simulink model "wlan_phy_rx_pmd"  (Simulink 7.8) (continued)
== system wlan_phy_rx_pmd/MAC Outputs == (continued)
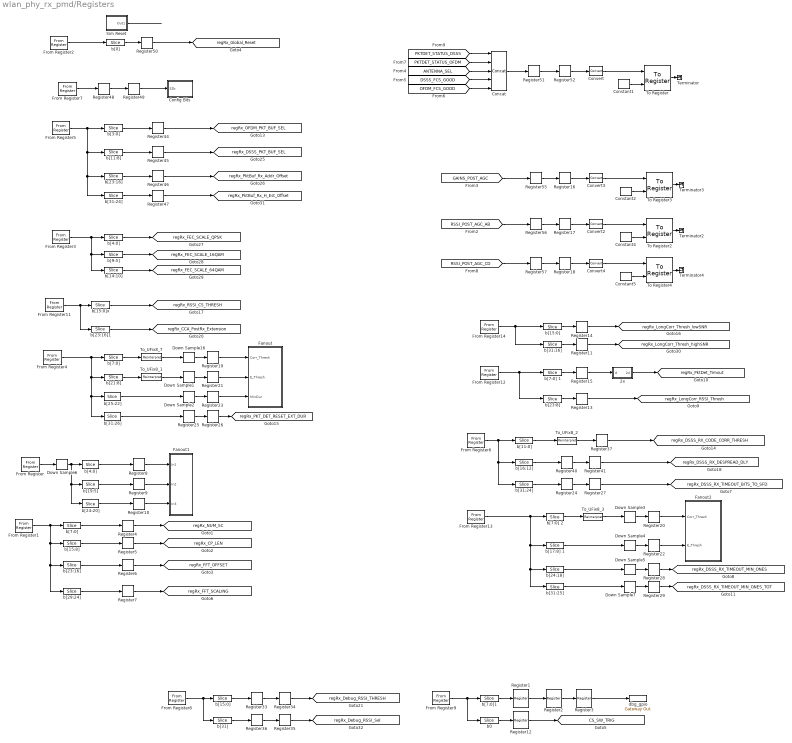
== system wlan_phy_rx_pmd/Registers ==
  " b[23:16] " : xbsIndex_r4/Slice  nbits=8  boolean_output=off  mode=Lower Bit Location + Width  bit1=0  base1=MSB of Input  bit0=16  base0=LSB of Input  dbl_ovrd=off  block_type=slice
      settings: block_version=VER_STRING_GOES_HERE
  " b[31:24]" : xbsIndex_r4/Slice  nbits=8  boolean_output=off  mode=Lower Bit Location + Width  bit1=0  base1=MSB of Input  bit0=24  base0=LSB of Input  dbl_ovrd=off  block_type=slice
      settings: block_version=VER_STRING_GOES_HERE
  "2x " : Subsystem
  Concat : xbsIndex_r4/Concat  num_inputs=5  dbl_ovrd=off  block_type=concat
      settings: block_version=8.2.02
  Config Bits : Subsystem
  Constant{1..2,4..5} (4 alike) : xbsIndex_r4/Constant  const=1  gui_display_data_type=Boolean  arith_type=Boolean  n_bits=30  bin_pt=0  preci_type=Single  exp_width=8  frac_width=24  explicit_period=on  period=1  equ=P=C  opselect=C  inp2=PCIN>>17  opr=+  inp1=P  carry=CIN  dbl_ovrd=off  block_type=constant
  Convert{,2..4} (4 alike) : xbsIndex_r4/Convert  gui_display_data_type=Fixed-point  arith_type=Unsigned  n_bits=32  bin_pt=0  float_type=Single  exp_bits=8  fraction_bits=24  quantization=Truncate  overflow=Wrap  en=off  latency=0  dbl_ovrd=off  pipeline=off  block_type=convert
  Down Sample{1..5,7,16} (7 alike) : xbsIndex_r4/Down Sample  sample_ratio=8  sample_phase="Last Value of Frame  (most efficient)"  en=off  latency=1  dbl_ovrd=off  block_type=dsamp
      settings: block_version=VER_STRING_GOES_HERE
  Down Sample6 : xbsIndex_r4/Down Sample  sample_ratio=16  sample_phase="Last Value of Frame  (most efficient)"  en=off  latency=1  dbl_ovrd=off  block_type=dsamp
      settings: block_version=VER_STRING_GOES_HERE
  Fanout : Subsystem
  Fanout1 : Subsystem
  Fanout2 : Subsystem
  From Register : xbsIndex_r4/From Register  shared_memory_name='PKTDET_RSSI_CONFIG'  init=REG_RX_PKTDET_RSSI_CONFIG  period=1  ownership=Locally owned and initialized  gui_display_data_type=Fixed-point  arith_type=Unsigned  n_bits=32  bin_pt=0  preci_type=Single  dbl_ovrd=off  block_type=fromreg
      settings: AttributesFormatString=<< %<shared_memory_name> >>  infoedit="Register block that reads data to a shared memory register.  Delay of one sample period."
  From Register1 : xbsIndex_r4/From Register  shared_memory_name='FFT_Config'  init=REG_RX_FFT_Config  period=1  ownership=Locally owned and initialized  gui_display_data_type=Fixed-point  arith_type=Unsigned  n_bits=32  bin_pt=0  preci_type=Single  dbl_ovrd=off  block_type=fromreg
      settings: AttributesFormatString=<< %<shared_memory_name> >>  infoedit="Register block that reads data to a shared memory register.  Delay of one sample period."
  From Register11 : xbsIndex_r4/From Register  shared_memory_name='PHY_CCA_CONFIG'  init=REG_RX_CCA_CONFIG  period=1  ownership=Locally owned and initialized  gui_display_data_type=Fixed-point  arith_type=Unsigned  n_bits=32  bin_pt=0  preci_type=Single  dbl_ovrd=off  block_type=fromreg
      settings: AttributesFormatString=<< %<shared_memory_name> >>  infoedit="Register block that reads data to a shared memory register.  Delay of one sample period."
  From Register12 : xbsIndex_r4/From Register  shared_memory_name='LTS_Corr_Config'  init=REG_RX_LTS_Corr_Confg  period=1  ownership=Locally owned and initialized  gui_display_data_type=Fixed-point  arith_type=Unsigned  n_bits=32  bin_pt=0  preci_type=Single  dbl_ovrd=off  block_type=fromreg
      settings: AttributesFormatString=<< %<shared_memory_name> >>  infoedit="Register block that reads data to a shared memory register.  Delay of one sample period."
  From Register13 : xbsIndex_r4/From Register  shared_memory_name='PKTDET_DSSS_CONFIG'  init=REG_RX_PktDet_DSSS_Config  period=1  ownership=Locally owned and initialized  gui_display_data_type=Fixed-point  arith_type=Unsigned  n_bits=32  bin_pt=0  preci_type=Single  dbl_ovrd=off  block_type=fromreg
      settings: AttributesFormatString=<< %<shared_memory_name> >>  infoedit="Register block that reads data to a shared memory register.  Delay of one sample period."
  From Register14 : xbsIndex_r4/From Register  shared_memory_name='LTS_Corr_Thresh'  init=REG_RX_LTS_Corr_Thresh  period=1  ownership=Locally owned and initialized  gui_display_data_type=Fixed-point  arith_type=Unsigned  n_bits=32  bin_pt=0  preci_type=Single  dbl_ovrd=off  block_type=fromreg
      settings: AttributesFormatString=<< %<shared_memory_name> >>  infoedit="Register block that reads data to a shared memory register.  Delay of one sample period."
  From Register2 : xbsIndex_r4/From Register  shared_memory_name='Control'  init=REG_RX_Control  period=1  ownership=Locally owned and initialized  gui_display_data_type=Fixed-point  arith_type=Unsigned  n_bits=32  bin_pt=0  preci_type=Single  dbl_ovrd=off  block_type=fromreg
      settings: AttributesFormatString=<< %<shared_memory_name> >>  infoedit="Register block that reads data to a shared memory register.  Delay of one sample period."
  From Register3 : xbsIndex_r4/From Register  shared_memory_name='FEC_CONFIG'  init=REG_RX_FEC_Config  period=1  ownership=Locally owned and initialized  gui_display_data_type=Fixed-point  arith_type=Unsigned  n_bits=32  bin_pt=0  preci_type=Single  dbl_ovrd=off  block_type=fromreg
      settings: AttributesFormatString=<< %<shared_memory_name> >>  infoedit="Register block that reads data to a shared memory register.  Delay of one sample period."
  From Register4 : xbsIndex_r4/From Register  shared_memory_name='PKTDET_AUTOCORR_CONFIG'  init=REG_RX_PktDet_AutoCorr_Config  period=1  ownership=Locally owned and initialized  gui_display_data_type=Fixed-point  arith_type=Unsigned  n_bits=32  bin_pt=0  preci_type=Single  dbl_ovrd=off  block_type=fromreg
      settings: AttributesFormatString=<< %<shared_memory_name> >>  infoedit="Register block that reads data to a shared memory register.  Delay of one sample period."
  From Register5 : xbsIndex_r4/From Register  shared_memory_name='PKT_BUF_SEL'  init=REG_RX_PktBuf_Sel  period=1  ownership=Locally owned and initialized  gui_display_data_type=Fixed-point  arith_type=Unsigned  n_bits=32  bin_pt=0  preci_type=Single  dbl_ovrd=off  block_type=fromreg
      settings: AttributesFormatString=<< %<shared_memory_name> >>  infoedit="Register block that reads data to a shared memory register.  Delay of one sample period."
  From Register6 : xbsIndex_r4/From Register  shared_memory_name='DSSS_RX_CONFIG'  init=REG_RX_DSSS_RX_CONFIG  period=1  ownership=Locally owned and initialized  gui_display_data_type=Fixed-point  arith_type=Unsigned  n_bits=32  bin_pt=0  preci_type=Single  dbl_ovrd=off  block_type=fromreg
      settings: AttributesFormatString=<< %<shared_memory_name> >>  infoedit="Register block that reads data to a shared memory register.  Delay of one sample period."
  From Register7 : xbsIndex_r4/From Register  shared_memory_name='CONFIG'  init=REG_RX_Config  period=1  ownership=Locally owned and initialized  gui_display_data_type=Fixed-point  arith_type=Unsigned  n_bits=32  bin_pt=0  preci_type=Single  dbl_ovrd=off  block_type=fromreg
      settings: AttributesFormatString=<< %<shared_memory_name> >>  infoedit="Register block that reads data to a shared memory register.  Delay of one sample period."
  From Register8 : xbsIndex_r4/From Register  shared_memory_name='RSSI_THRESH'  init=600  period=1  ownership=Locally owned and initialized  gui_display_data_type=Fixed-point  arith_type=Unsigned  n_bits=32  bin_pt=0  preci_type=Single  dbl_ovrd=off  block_type=fromreg
      settings: AttributesFormatString=<< %<shared_memory_name> >>  infoedit="Register block that reads data to a shared memory register.  Delay of one sample period."
  From Register9 : xbsIndex_r4/From Register  shared_memory_name='DEBUG_GPIO'  init=0  period=1  ownership=Locally owned and initialized  gui_display_data_type=Fixed-point  arith_type=Unsigned  n_bits=32  bin_pt=0  preci_type=Single  dbl_ovrd=off  block_type=fromreg
      settings: AttributesFormatString=<< %<shared_memory_name> >>  infoedit="Register block that reads data to a shared memory register.  Delay of one sample period."
  Register{1..29,33..37,40..41,44..52,55..57} (48 alike) : xbsIndex_r4/Register  init=0  rst=off  en=off  dbl_ovrd=off  block_type=register
  Sim Reset : Subsystem
  Terminator{,2..4} (4 alike) : Terminator
  To Register : xbsIndex_r4/To Register  shared_memory_name='Status'  init=0  ownership=Locally owned and initialized  explicit_data_type=on  gui_display_data_type=Fixed-point  arith_type=Unsigned  n_bits=32  bin_pt=0  preci_type=Single  dbl_ovrd=off  block_type=toreg
      settings: AttributesFormatString=<< %<shared_memory_name> >>  infoedit="Register block that writes data to a shared memory register.  Delay of one sample period."
  To Register2 : xbsIndex_r4/To Register  shared_memory_name='RX_PKT_RSSI_AB'  init=0  ownership=Locally owned and initialized  explicit_data_type=on  gui_display_data_type=Fixed-point  arith_type=Unsigned  n_bits=32  bin_pt=0  preci_type=Single  dbl_ovrd=off  block_type=toreg
      settings: AttributesFormatString=<< %<shared_memory_name> >>  infoedit="Register block that writes data to a shared memory register.  Delay of one sample period."
  To Register3 : xbsIndex_r4/To Register  shared_memory_name='RX_PKT_AGC_GAINS'  init=0  ownership=Locally owned and initialized  explicit_data_type=on  gui_display_data_type=Fixed-point  arith_type=Unsigned  n_bits=32  bin_pt=0  preci_type=Single  dbl_ovrd=off  block_type=toreg
      settings: AttributesFormatString=<< %<shared_memory_name> >>  infoedit="Register block that writes data to a shared memory register.  Delay of one sample period."
  To Register4 : xbsIndex_r4/To Register  shared_memory_name='RX_PKT_RSSI_CD'  init=0  ownership=Locally owned and initialized  explicit_data_type=on  gui_display_data_type=Fixed-point  arith_type=Unsigned  n_bits=32  bin_pt=0  preci_type=Single  dbl_ovrd=off  block_type=toreg
      settings: AttributesFormatString=<< %<shared_memory_name> >>  infoedit="Register block that writes data to a shared memory register.  Delay of one sample period."
  To_UFix8_1 : xbsIndex_r4/Reinterpret  force_arith_type=on  arith_type=Unsigned  force_bin_pt=on  bin_pt=8  block_type=cast
      settings: block_version=VER_STRING_GOES_HERE
  To_UFix8_2 : xbsIndex_r4/Reinterpret  force_arith_type=on  arith_type=Unsigned  force_bin_pt=on  bin_pt=4  block_type=cast
      settings: block_version=VER_STRING_GOES_HERE
  To_UFix8_3 : xbsIndex_r4/Reinterpret  force_arith_type=on  arith_type=Unsigned  force_bin_pt=on  bin_pt=6  block_type=cast
      settings: block_version=VER_STRING_GOES_HERE
  To_UFix8_7 : xbsIndex_r4/Reinterpret  force_arith_type=on  arith_type=Unsigned  force_bin_pt=on  bin_pt=8  block_type=cast
      settings: block_version=VER_STRING_GOES_HERE
  b0 : xbsIndex_r4/Slice  nbits=1  boolean_output=off  mode=Lower Bit Location + Width  bit1=0  base1=MSB of Input  bit0=0  base0=LSB of Input  dbl_ovrd=off  block_type=slice
      settings: block_version=VER_STRING_GOES_HERE
  b[0] : xbsIndex_r4/Slice  nbits=1  boolean_output=off  mode=Lower Bit Location + Width  bit1=0  base1=MSB of Input  bit0=0  base0=LSB of Input  dbl_ovrd=off  block_type=slice
      settings: block_version=VER_STRING_GOES_HERE
  "b[11:0]   " : xbsIndex_r4/Slice  nbits=12  boolean_output=off  mode=Lower Bit Location + Width  bit1=0  base1=MSB of Input  bit0=0  base0=LSB of Input  dbl_ovrd=off  block_type=slice
      settings: block_version=VER_STRING_GOES_HERE
  b[11:8] : xbsIndex_r4/Slice  nbits=4  boolean_output=off  mode=Lower Bit Location + Width  bit1=0  base1=MSB of Input  bit0=8  base0=LSB of Input  dbl_ovrd=off  block_type=slice
      settings: block_version=VER_STRING_GOES_HERE
  b[14:10] : xbsIndex_r4/Slice  nbits=5  boolean_output=off  mode=Lower Bit Location + Width  bit1=0  base1=MSB of Input  bit0=10  base0=LSB of Input  dbl_ovrd=off  block_type=slice
      settings: block_version=VER_STRING_GOES_HERE
  b[15:0] : xbsIndex_r4/Slice  nbits=16  boolean_output=off  mode=Lower Bit Location + Width  bit1=0  base1=MSB of Input  bit0=0  base0=LSB of Input  dbl_ovrd=off  block_type=slice
      settings: block_version=VER_STRING_GOES_HERE
  "b[15:0]    " : xbsIndex_r4/Slice  nbits=16  boolean_output=off  mode=Lower Bit Location + Width  bit1=0  base1=MSB of Input  bit0=0  base0=LSB of Input  dbl_ovrd=off  block_type=slice
      settings: block_version=VER_STRING_GOES_HERE
  b[15:0]x : xbsIndex_r4/Slice  nbits=16  boolean_output=off  mode=Lower Bit Location + Width  bit1=0  base1=MSB of Input  bit0=0  base0=LSB of Input  dbl_ovrd=off  block_type=slice
      settings: block_version=VER_STRING_GOES_HERE
  b[15:8] : xbsIndex_r4/Slice  nbits=8  boolean_output=off  mode=Lower Bit Location + Width  bit1=0  base1=MSB of Input  bit0=8  base0=LSB of Input  dbl_ovrd=off  block_type=slice
      settings: block_version=VER_STRING_GOES_HERE
  "b[16:12]   " : xbsIndex_r4/Slice  nbits=5  boolean_output=off  mode=Lower Bit Location + Width  bit1=0  base1=MSB of Input  bit0=12  base0=LSB of Input  dbl_ovrd=off  block_type=slice
      settings: block_version=VER_STRING_GOES_HERE
  b[17:8] 1 : xbsIndex_r4/Slice  nbits=10  boolean_output=off  mode=Lower Bit Location + Width  bit1=0  base1=MSB of Input  bit0=8  base0=LSB of Input  dbl_ovrd=off  block_type=slice
      settings: block_version=VER_STRING_GOES_HERE
  b[19:5] : xbsIndex_r4/Slice  nbits=15  boolean_output=off  mode=Lower Bit Location + Width  bit1=0  base1=MSB of Input  bit0=5  base0=LSB of Input  dbl_ovrd=off  block_type=slice
      settings: block_version=VER_STRING_GOES_HERE
  "b[21:8] " : xbsIndex_r4/Slice  nbits=14  boolean_output=off  mode=Lower Bit Location + Width  bit1=0  base1=MSB of Input  bit0=8  base0=LSB of Input  dbl_ovrd=off  block_type=slice
      settings: block_version=VER_STRING_GOES_HERE
  b[23:16] : xbsIndex_r4/Slice  nbits=8  boolean_output=off  mode=Lower Bit Location + Width  bit1=0  base1=MSB of Input  bit0=16  base0=LSB of Input  dbl_ovrd=off  block_type=slice
      settings: block_version=VER_STRING_GOES_HERE
  b[23:16]1 : xbsIndex_r4/Slice  nbits=8  boolean_output=off  mode=Lower Bit Location + Width  bit1=0  base1=MSB of Input  bit0=16  base0=LSB of Input  dbl_ovrd=off  block_type=slice
      settings: block_version=VER_STRING_GOES_HERE
  b[23:8] : xbsIndex_r4/Slice  nbits=16  boolean_output=off  mode=Lower Bit Location + Width  bit1=0  base1=MSB of Input  bit0=8  base0=LSB of Input  dbl_ovrd=off  block_type=slice
      settings: block_version=VER_STRING_GOES_HERE
  b[24:18] : xbsIndex_r4/Slice  nbits=7  boolean_output=off  mode=Lower Bit Location + Width  bit1=0  base1=MSB of Input  bit0=18  base0=LSB of Input  dbl_ovrd=off  block_type=slice
      settings: block_version=VER_STRING_GOES_HERE
  b[24:20] : xbsIndex_r4/Slice  nbits=5  boolean_output=off  mode=Lower Bit Location + Width  bit1=0  base1=MSB of Input  bit0=20  base0=LSB of Input  dbl_ovrd=off  block_type=slice
      settings: block_version=VER_STRING_GOES_HERE
  "b[25:22] " : xbsIndex_r4/Slice  nbits=4  boolean_output=off  mode=Lower Bit Location + Width  bit1=0  base1=MSB of Input  bit0=22  base0=LSB of Input  dbl_ovrd=off  block_type=slice
      settings: block_version=VER_STRING_GOES_HERE
  b[29:24] : xbsIndex_r4/Slice  nbits=6  boolean_output=off  mode=Lower Bit Location + Width  bit1=0  base1=MSB of Input  bit0=24  base0=LSB of Input  dbl_ovrd=off  block_type=slice
      settings: block_version=VER_STRING_GOES_HERE
  b[31:16] : xbsIndex_r4/Slice  nbits=16  boolean_output=off  mode=Lower Bit Location + Width  bit1=0  base1=MSB of Input  bit0=16  base0=LSB of Input  dbl_ovrd=off  block_type=slice
      settings: block_version=VER_STRING_GOES_HERE
  b[31:24] : xbsIndex_r4/Slice  nbits=8  boolean_output=off  mode=Lower Bit Location + Width  bit1=0  base1=MSB of Input  bit0=24  base0=LSB of Input  dbl_ovrd=off  block_type=slice
      settings: block_version=VER_STRING_GOES_HERE
  b[31:25] : xbsIndex_r4/Slice  nbits=7  boolean_output=off  mode=Lower Bit Location + Width  bit1=0  base1=MSB of Input  bit0=25  base0=LSB of Input  dbl_ovrd=off  block_type=slice
      settings: block_version=VER_STRING_GOES_HERE
  b[31:26] : xbsIndex_r4/Slice  nbits=6  boolean_output=off  mode=Lower Bit Location + Width  bit1=0  base1=MSB of Input  bit0=26  base0=LSB of Input  dbl_ovrd=off  block_type=slice
      settings: block_version=VER_STRING_GOES_HERE
  b[31] : xbsIndex_r4/Slice  nbits=1  boolean_output=off  mode=Lower Bit Location + Width  bit1=0  base1=MSB of Input  bit0=31  base0=LSB of Input  dbl_ovrd=off  block_type=slice
      settings: block_version=VER_STRING_GOES_HERE
  b[3:0] : xbsIndex_r4/Slice  nbits=4  boolean_output=off  mode=Lower Bit Location + Width  bit1=0  base1=MSB of Input  bit0=0  base0=LSB of Input  dbl_ovrd=off  block_type=slice
      settings: block_version=VER_STRING_GOES_HERE
  b[4:0] : xbsIndex_r4/Slice  nbits=5  boolean_output=off  mode=Lower Bit Location + Width  bit1=0  base1=MSB of Input  bit0=0  base0=LSB of Input  dbl_ovrd=off  block_type=slice
      settings: block_version=VER_STRING_GOES_HERE
  "b[4:0]  " : xbsIndex_r4/Slice  nbits=5  boolean_output=off  mode=Lower Bit Location + Width  bit1=0  base1=MSB of Input  bit0=0  base0=LSB of Input  dbl_ovrd=off  block_type=slice
      settings: block_version=VER_STRING_GOES_HERE
  b[7:0] : xbsIndex_r4/Slice  nbits=8  boolean_output=off  mode=Lower Bit Location + Width  bit1=0  base1=MSB of Input  bit0=0  base0=LSB of Input  dbl_ovrd=off  block_type=slice
      settings: block_version=VER_STRING_GOES_HERE
  "b[7:0] " : xbsIndex_r4/Slice  nbits=8  boolean_output=off  mode=Lower Bit Location + Width  bit1=0  base1=MSB of Input  bit0=0  base0=LSB of Input  dbl_ovrd=off  block_type=slice
      settings: block_version=VER_STRING_GOES_HERE
  b[7:0] 1 : xbsIndex_r4/Slice  nbits=8  boolean_output=off  mode=Lower Bit Location + Width  bit1=0  base1=MSB of Input  bit0=0  base0=LSB of Input  dbl_ovrd=off  block_type=slice
      settings: block_version=VER_STRING_GOES_HERE
  b[7:0] 2 : xbsIndex_r4/Slice  nbits=8  boolean_output=off  mode=Lower Bit Location + Width  bit1=0  base1=MSB of Input  bit0=0  base0=LSB of Input  dbl_ovrd=off  block_type=slice
      settings: block_version=VER_STRING_GOES_HERE
  b[7:0]1 : xbsIndex_r4/Slice  nbits=8  boolean_output=off  mode=Lower Bit Location + Width  bit1=0  base1=MSB of Input  bit0=0  base0=LSB of Input  dbl_ovrd=off  block_type=slice
      settings: block_version=VER_STRING_GOES_HERE
  b[9:5] : xbsIndex_r4/Slice  nbits=5  boolean_output=off  mode=Lower Bit Location + Width  bit1=0  base1=MSB of Input  bit0=5  base0=LSB of Input  dbl_ovrd=off  block_type=slice
      settings: block_version=VER_STRING_GOES_HERE
  dbg_gpio : xbsIndex_r4/Gateway Out  inherit_from_input=off  hdl_port=on  timing_constraint=None  locs_specified=off  LOCs={}  UseAsDAC=off  DACChannel='1'  block_type=gatewayout
  tag scope: CS_SW_TRIG=global, regRx_CCA_PostRx_Extension=global, regRx_CP_LEN=global, regRx_DSSS_PKT_BUF_SEL=global, regRx_DSSS_RX_CODE_CORR_THRESH=global, regRx_DSSS_RX_DESPREAD_DLY=global, regRx_DSSS_RX_TIMEOUT_BITS_TO_SFD=global, regRx_DSSS_RX_TIMEOUT_MIN_ONES=global, regRx_DSSS_RX_TIMEOUT_MIN_ONES_TOT=global, regRx_Debug_RSSI_Sel=global, regRx_Debug_RSSI_THRESH=global, regRx_FEC_SCALE_16QAM=global, regRx_FEC_SCALE_64QAM=global, regRx_FEC_SCALE_QPSK=global, regRx_FFT_OFFSET=global, regRx_FFT_SCALING=global, regRx_Global_Reset=global, regRx_LongCorr_RSSI_Thresh=global, regRx_LongCorr_Thresh_highSNR=global, regRx_LongCorr_Thresh_lowSNR=global, regRx_NUM_SC=global, regRx_OFDM_PKT_BUF_SEL=global, regRx_PKT_DET_RESET_EXT_DUR=global, regRx_PktBuf_Rx_Addr_Offset=global, regRx_PktBuf_Rx_H_Est_Offset=global, regRx_PktDet_Timout=global, regRx_RSSI_CS_THRESH=global
  wires:
  b[31:16]/1 -> Register11/1
  From Register1/1 -> b[7:0]/1, b[15:8]/1, b[23:16]/1, b[29:24]/1
  b[7:0]/1 -> Register4/1
  b[15:8]/1 -> Register5/1
  b[23:16]/1 -> Register6/1
  From Register2/1 -> b[0]/1
  b[0]/1 -> Register50/1
  From Register3/1 -> "b[4:0]  "/1, b[9:5]/1, b[14:10]/1
  b[29:24]/1 -> Register7/1
  [DSSS_FCS_GOOD] -> Concat/4
  [OFDM_FCS_GOOD] -> Concat/5
  Constant1/1 -> To Register/2
  To Register/1 -> Terminator/1
  From Register4/1 -> "b[7:0] "/1, "b[21:8] "/1, "b[25:22] "/1, b[31:26]/1
  "b[7:0] "/1 -> To_UFix8_7/1
  "b[21:8] "/1 -> To_UFix8_1/1
  To_UFix8_7/1 -> Down Sample16/1
  To_UFix8_1/1 -> Down Sample1/1
  Down Sample16/1 -> Register19/1
  Down Sample1/1 -> Register21/1
  b[15:0]/1 -> Register14/1
  "b[25:22] "/1 -> Down Sample2/1
  Down Sample2/1 -> Register23/1
  Sim Reset/1(Out1) -> ?
  From Register5/1 -> b[3:0]/1, b[11:8]/1, " b[23:16] "/1, " b[31:24]"/1
  b[3:0]/1 -> Register44/1
  From Register6/1 -> "b[11:0]   "/1, "b[16:12]   "/1, b[31:24]/1
  "b[11:0]   "/1 -> To_UFix8_2/1
  To_UFix8_2/1 -> Register37/1
  From Register8/1 -> "b[15:0]    "/1, b[31]/1
  "b[15:0]    "/1 -> Register33/1
  From Register9/1 -> b[7:0]1/1, b0/1
  b[7:0]1/1 -> Register1/1
  Register1/1 -> Register2/1
  Register2/1 -> Register3/1
  Register3/1 -> dbg_gpio/1
  b[4:0]/1 -> Register8/1
  Down Sample6/1 -> b[4:0]/1, b[19:5]/1, b[24:20]/1
  b[19:5]/1 -> Register9/1
  From Register/1 -> Down Sample6/1
  b[24:20]/1 -> Register10/1
  From Register7/1 -> Register48/1
  Register4/1 -> [regRx_NUM_SC]
  Register5/1 -> [regRx_CP_LEN]
  Register6/1 -> [regRx_FFT_OFFSET]
  Register7/1 -> [regRx_FFT_SCALING]
  Register8/1 -> Fanout1/1(In1)
  Register9/1 -> Fanout1/2(In2)
  Register10/1 -> Fanout1/3(In3)
  Register11/1 -> [regRx_LongCorr_Thresh_highSNR]
  b[31:26]/1 -> Register25/1
  From Register11/1 -> b[15:0]x/1, b[23:16]1/1
  b[15:0]x/1 -> [regRx_RSSI_CS_THRESH]
  b[23:16]1/1 -> [regRx_CCA_PostRx_Extension]
  b[11:8]/1 -> Register45/1
  Convert/1 -> To Register/1
  " b[23:16] "/1 -> Register46/1
  "b[4:0]  "/1 -> [regRx_FEC_SCALE_QPSK]
  b[9:5]/1 -> [regRx_FEC_SCALE_16QAM]
  b[14:10]/1 -> [regRx_FEC_SCALE_64QAM]
  b0/1 -> Register12/1
  Register12/1 -> [CS_SW_TRIG]
  Constant4/1 -> To Register2/2
  To Register2/1 -> Terminator2/1
  Convert2/1 -> To Register2/1
  Register13/1 -> [regRx_LongCorr_RSSI_Thresh]
  b[23:8]/1 -> Register13/1
  Register14/1 -> [regRx_LongCorr_Thresh_lowSNR]
  From Register14/1 -> b[15:0]/1, b[31:16]/1
  From Register12/1 -> b[7:0] 1/1, b[23:8]/1
  "2x "/1(2d) -> [regRx_PktDet_Timout]
  Register15/1 -> "2x "/1(d)
  b[7:0] 1/1 -> Register15/1
  " b[31:24]"/1 -> Register47/1
  [RSSI_POST_AGC_AB] -> Register56/1
  [ANTENNA_SEL] -> Concat/3
  Constant2/1 -> To Register3/2
  To Register3/1 -> Terminator3/1
  Convert3/1 -> To Register3/1
  [GAINS_POST_AGC] -> Register55/1
  Register16/1 -> Convert3/1
  Register17/1 -> Convert2/1
  b[31]/1 -> Register36/1
  Concat/1 -> Register51/1
  [PKTDET_STATUS_OFDM] -> Concat/2
  Constant5/1 -> To Register4/2
  To Register4/1 -> Terminator4/1
  Convert4/1 -> To Register4/1
  Register18/1 -> Convert4/1
  [RSSI_POST_AGC_CD] -> Register57/1
  Register19/1 -> Fanout/1(Corr_Thresh)
  Register21/1 -> Fanout/2(E_Thresh)
  Register23/1 -> Fanout/3(MinDur)
  Register33/1 -> Register34/1
  Register34/1 -> [regRx_Debug_RSSI_THRESH]
  Register35/1 -> [regRx_Debug_RSSI_Sel]
  Register36/1 -> Register35/1
  Register37/1 -> [regRx_DSSS_RX_CODE_CORR_THRESH]
  Register40/1 -> Register41/1
  Register41/1 -> [regRx_DSSS_RX_DESPREAD_DLY]
  Register44/1 -> [regRx_OFDM_PKT_BUF_SEL]
  Register45/1 -> [regRx_DSSS_PKT_BUF_SEL]
  Register46/1 -> [regRx_PktBuf_Rx_Addr_Offset]
  Register47/1 -> [regRx_PktBuf_Rx_H_Est_Offset]
  Register48/1 -> Register49/1
  Register49/1 -> Config Bits/1(32b)
  Register50/1 -> [regRx_Global_Reset]
  Register51/1 -> Register52/1
  Register52/1 -> Convert/1
  Register55/1 -> Register16/1
  Register56/1 -> Register17/1
  Register57/1 -> Register18/1
  Register25/1 -> Register26/1
  Register26/1 -> [regRx_PKT_DET_RESET_EXT_DUR]
  From Register13/1 -> b[7:0] 2/1, b[17:8] 1/1, b[24:18]/1, b[31:25]/1
  b[7:0] 2/1 -> To_UFix8_3/1
  To_UFix8_3/1 -> Down Sample3/1
  Down Sample3/1 -> Register20/1
  Register20/1 -> Fanout2/1(Corr_Thresh)
  b[17:8] 1/1 -> Down Sample4/1
  Down Sample4/1 -> Register22/1
  Register22/1 -> Fanout2/2(E_Thresh)
  [PKTDET_STATUS_DSSS] -> Concat/1
  "b[16:12]   "/1 -> Register40/1
  b[31:24]/1 -> Register24/1
  Register24/1 -> Register27/1
  Register27/1 -> [regRx_DSSS_RX_TIMEOUT_BITS_TO_SFD]
  b[24:18]/1 -> Down Sample5/1
  Down Sample5/1 -> Register28/1
  Register28/1 -> [regRx_DSSS_RX_TIMEOUT_MIN_ONES]
  b[31:25]/1 -> Down Sample7/1
  Down Sample7/1 -> Register29/1
  Register29/1 -> [regRx_DSSS_RX_TIMEOUT_MIN_ONES_TOT]

== system wlan_phy_rx_pmd/Resets ==
  Convert1 : xbsIndex_r4/Convert  gui_display_data_type=Boolean  arith_type=Boolean  n_bits=16  bin_pt=14  float_type=Single  exp_bits=8  fraction_bits=24  quantization=Truncate  overflow=Wrap  en=off  latency=0  dbl_ovrd=off  pipeline=off  block_type=convert
  Pulse Generator : DiscretePulseGenerator  Period=1e9  PulseWidth=8  PhaseDelay=100
  tag scope: RX_GLOBAL_RESET=global
  wires:
  [regRx_Global_Reset] -> Convert1/1
  Convert1/1 -> [RX_GLOBAL_RESET]

== system wlan_phy_rx_pmd/Sync & Antenna Sel ==
  Rx I : Inport
  Rx Q : Inport  Port=2
  IQ Valid : Inport  Port=3
  RSSI : Inport  Port=4
  OFDM Reset : Inport  Port=5
  ADC/Corr : Scope  NumInputPorts=10
      settings: TimeRange=16508  YMin=0.95~-1~-1~0.95~-1~-1~-1~-1~-1~-1  YMax=1.05~1~1~1.05~1~1~1~1~1~1  SaveName=ScopeData34
  Antenna Selection : Subsystem
  Debug  Outputs : Subsystem
  From Workspace1 : FromWorkspace  VariableName=samps_pkt_det  SampleTime=paylod_vec_samp_time  Interpolate=off  OutputAfterFinalValue=Setting to zero
  Gateway Out{,1..4} (5 alike) : xbsIndex_r4/Gateway Out  inherit_from_input=off  hdl_port=off  timing_constraint=None  locs_specified=off  LOCs={}  UseAsDAC=off  DACChannel='1'  block_type=gatewayout
  LTS Correlation : Subsystem
  PHY CCA : Subsystem
  Pkt Det : Subsystem
  Pkt Det AGC Ctrl : Subsystem
  Resets : Subsystem
  " Pkt Det DSSS" : Outport
  " Rx I" : Outport  Port=2
  " Rx Q" : Outport  Port=3
  " IQ Valid" : Outport  Port=4
  " Pkt Det OFDM" : Outport  Port=5
  LTS Sync : Outport  Port=6
  wires:
  LTS Correlation/1(Det) -> LTS Sync/1, PHY CCA/1(LTS Sync), Debug  Outputs/1(LTS)
  LTS Correlation/2(Timeout) -> PHY CCA/2(LTS Timeout), Resets/3(LTS Timeout), Debug  Outputs/2(LTS Timeout), Gateway Out2/1
  From Workspace1/1 -> ADC/Corr/10  "Capt Pkt Det"
  Gateway Out2/1 -> ADC/Corr/3  "LTS TO"
  Gateway Out1/1 -> ADC/Corr/2  "Pkt Done"
  Gateway Out/1 -> ADC/Corr/1  "Pkt Det OFDM"
  Pkt Det/1(Det OFDM) -> Antenna Selection/1(Pkt Det), LTS Correlation/5(En), PHY CCA/4(Pkt Det), Debug  Outputs/3(Det OFDM), Gateway Out/1, " Pkt Det OFDM"/1, Pkt Det AGC Ctrl/1(Det OFDM)
  Resets/1(" OFDM Reset") -> Antenna Selection/6(Reset), LTS Correlation/6(Reset), PHY CCA/6(Reset), Gateway Out1/1, Pkt Det/1(Reset OFDM)
  OFDM Reset/1 -> Resets/1(OFDM Reset)
  IQ Valid/1 -> Pkt Det/5(IQ Valid), Antenna Selection/4(IQ Valid)
  RSSI/1 -> Pkt Det/6(RSSI Vec), Antenna Selection/5(RSSI Vec), PHY CCA/5(Avg RSSI Vec)
  Rx I/1 -> Pkt Det/3(Rx I Vec), Antenna Selection/2(Rx I Vec)
  Rx Q/1 -> Pkt Det/4(Rx Q Vec), Antenna Selection/3(Rx Q Vec)
  Antenna Selection/1(Rx I) -> " Rx I"/1, LTS Correlation/1(rx_I)
  Antenna Selection/2(" Rx Q") -> " Rx Q"/1, LTS Correlation/2(rx_Q)
  Antenna Selection/3(" IQ Valid") -> " IQ Valid"/1, LTS Correlation/3(rx_IQ_valid), PHY CCA/3(IQ Valid)
  Antenna Selection/4(RSSI) -> LTS Correlation/4(RSSI), Gateway Out3/1  "Avg RSSI"
  Gateway Out3/1 -> ADC/Corr/9
  Pkt Det/2(Det DSSS) -> Gateway Out4/1, " Pkt Det DSSS"/1, Pkt Det AGC Ctrl/2(Det DSSS), Debug  Outputs/4(Det DSSS)
  Gateway Out4/1 -> ADC/Corr/4  "Pkt Det DSSS"
  Resets/2(DSSS Reset) -> Pkt Det/2(Reset DSSS)
  [DSSS_RX_RESET] -> Resets/2("DSSS Reset ")
